simulink library "lib_all"  (Simulink 7.6)
settings: Solver=ode45  SolverMode=SingleTasking  StartTime=0.0  StopTime=10.0  RelTol=1e-3
values from neighbouring files (symbols this model uses):
  map_lib_03 = [6.8679 7.0159 7.4571 8.1849 9.1892 10.456 11.97 13.71 15.655 17.781 20.063 22.474 ... (803 elements, 73x11)]    [scr_simdata.mat]    (also in scr_simdata_all.mat)
  map_lib_06 = [2.5784 2.6727 2.9588 3.4453 4.1444 5.068 6.226 7.6242 9.2634 11.139 13.24 15.552 ... (803 elements, 73x11)]    [scr_simdata.mat]    (also in scr_simdata_all.mat)
  map_lib_09 = [0.0025784 0.0026727 0.0029588 0.0034453 0.0041444 0.005068 0.006226 0.0076242 0.0092634 0.011139 0.01324 0.015552 ... (803 elements, 73x11)]    [scr_simdata.mat]    (also in scr_simdata_all.mat)
  map_lib_10 = [2.74 2.75 2.76 2.77 2.78 2.79 2.8 2.81 2.82 2.83 2.84 2.85 ... (81 elements, 1x81)]    [scr_simdata.mat]    (also in scr_simdata_all.mat)
  map_lib_11 = [1.1983 1.2027 1.207 1.2113 1.2156 1.2198 1.2241 1.2283 1.2326 1.2368 1.241 1.2451 ... (81 elements, 1x81)]    [scr_simdata.mat]    (also in scr_simdata_all.mat)
  map_lib_12 = [2.74 2.75 2.76 2.77 2.78 2.79 2.8 2.81 2.82 2.83 2.84 2.85 ... (81 elements, 1x81)]    [scr_simdata.mat]    (also in scr_simdata_all.mat)
  map_lib_13 = [1.74 1.75 1.76 1.77 1.78 1.79 1.8 1.81 1.82 1.83 1.84 1.85 ... (81 elements, 1x81)]    [scr_simdata.mat]    (also in scr_simdata_all.mat)
  map_lib_16 = [0.00048828125 1.6159 1.8962 2.0821 2.2251 2.3426 2.4433 2.5318 2.611 2.683 2.749 2.8101 ... (1419 elements, 129x11)]    [scr_simdata.mat]    (also in scr_simdata_all.mat)
  map_mr2_01 = [0 800 1600 2400 3200 4000]    [scr_simdata.mat]    (also in scr_simdata_all.mat)
  map_mr2_02 = [1.32 1.32 1.33 1.34 1.36 1.38]    [scr_simdata.mat]    (also in scr_simdata_all.mat)
  map_mr2_03 = [0 800 1600 2400 3200 4000]    [scr_simdata.mat]    (also in scr_simdata_all.mat)
  map_mr2_04 = [1.32 1.32 1.33 1.34 1.36 1.38]    [scr_simdata.mat]    (also in scr_simdata_all.mat)
  map_mr2_05 = [0 800 1600 2400 3200 4000]    [scr_simdata.mat]    (also in scr_simdata_all.mat)
  map_mr2_06 = [1.32 1.32 1.33 1.34 1.36 1.38]    [scr_simdata.mat]    (also in scr_simdata_all.mat)
  Parameter1 = 6    [scr_simdata.mat]    (also in scr_simdata_all.mat)
  Parameter101 = 30    [scr_simdata.mat]    (also in scr_simdata_all.mat)
  Parameter102 = 12    [scr_simdata.mat]    (also in scr_simdata_all.mat)
  Parameter103 = -240    [scr_simdata.mat]    (also in scr_simdata_all.mat)
  Parameter108 = 48    [scr_simdata.mat]    (also in scr_simdata_all.mat)
  Parameter120 = 16    [scr_simdata.mat]    (also in scr_simdata_all.mat)
  Parameter121 = 87    [scr_simdata.mat]    (also in scr_simdata_all.mat)
  Parameter123 = 78    [scr_simdata.mat]    (also in scr_simdata_all.mat)
  Parameter124 = 60    [scr_simdata.mat]    (also in scr_simdata_all.mat)
  Parameter125 = -480    [scr_simdata.mat]    (also in scr_simdata_all.mat)
  Parameter130 = 96    [scr_simdata.mat]    (also in scr_simdata_all.mat)
  Parameter131 = 3    [scr_simdata.mat]    (also in scr_simdata_all.mat)
  Parameter144 = 3    [scr_simdata.mat]    (also in scr_simdata_all.mat)
  Parameter18 = 128    [scr_simdata.mat]    (also in scr_simdata_all.mat)
  Parameter19 = 16    [scr_simdata.mat]    (also in scr_simdata_all.mat)
  Parameter2 = 12    [scr_simdata.mat]    (also in scr_simdata_all.mat)
  Parameter20 = 7    [scr_simdata.mat]    (also in scr_simdata_all.mat)
  Parameter21 = 4    [scr_simdata.mat]    (also in scr_simdata_all.mat)
  Parameter3 = 18    [scr_simdata.mat]    (also in scr_simdata_all.mat)
  Parameter32 = 1    [scr_simdata.mat]    (also in scr_simdata_all.mat)
  Parameter34 = 24    [scr_simdata.mat]    (also in scr_simdata_all.mat)
  Parameter4 = 138    [scr_simdata.mat]    (also in scr_simdata_all.mat)
  Parameter42 = 48    [scr_simdata.mat]    (also in scr_simdata_all.mat)
  Parameter5 = 135    [scr_simdata.mat]    (also in scr_simdata_all.mat)
  Parameter55 = 0    [scr_simdata.mat]    (also in scr_simdata_all.mat)
  Parameter56 = 0    [scr_simdata.mat]    (also in scr_simdata_all.mat)
  Parameter57 = 1    [scr_simdata.mat]    (also in scr_simdata_all.mat)
  Parameter58 = 1    [scr_simdata.mat]    (also in scr_simdata_all.mat)
  Parameter59 = 2    [scr_simdata.mat]    (also in scr_simdata_all.mat)
  Parameter6 = 132    [scr_simdata.mat]    (also in scr_simdata_all.mat)
  Parameter60 = 2    [scr_simdata.mat]    (also in scr_simdata_all.mat)
  Parameter63 = 144    [scr_simdata.mat]    (also in scr_simdata_all.mat)
  Parameter7 = 126    [scr_simdata.mat]    (also in scr_simdata_all.mat)
  Parameter76 = 64    [scr_simdata.mat]    (also in scr_simdata_all.mat)
  Parameter77 = 135    [scr_simdata.mat]    (also in scr_simdata_all.mat)
  Parameter79 = 126    [scr_simdata.mat]    (also in scr_simdata_all.mat)
  Parameter80 = 108    [scr_simdata.mat]    (also in scr_simdata_all.mat)
  Parameter81 = 0    [scr_simdata.mat]    (also in scr_simdata_all.mat)
  Parameter86 = 0    [scr_simdata.mat]    (also in scr_simdata_all.mat)
  Parameter98 = 112    [scr_simdata.mat]    (also in scr_simdata_all.mat)
  Parameter99 = 39    [scr_simdata.mat]    (also in scr_simdata_all.mat)
undefined symbols (not defined in the file or in its folder): cylvolkp_hih_map2h, cylvolkp_map2h, pk1k_map2h
libraries: simulink x2
(part 23 of 36)

== system lib_all/SubSystem800/SubSystem819/SubSystem846/SubSystem854/SubSystem857 ==
  note: ***編集禁止***
  In1 : Inport
  In2 : Inport  Port=2
  RO1 : RelationalOperator  Operator===  InputSameDT=on
  single1 : DataTypeConversion  OutDataTypeStr=single  SaturateOnIntegerOverflow=on  RndMeth=Zero
  single2 : DataTypeConversion  OutDataTypeStr=single  SaturateOnIntegerOverflow=on  RndMeth=Zero
  Out1 : Outport
  wires:
  RO1/1 -> Out1/1
  In2/1 -> single2/1
  In1/1 -> single1/1
  single1/1 -> RO1/1
  single2/1 -> RO1/2

== system lib_all/SubSystem800/SubSystem819/SubSystem846/SubSystem854/SubSystem858 ==
  Block2180 : Inport
  Block2181 : Inport  Port=2
  Block2182 : EnablePort
  Block2183 : Demux  Outputs=2
  Block2184 : Mux  Inputs=2
  SubSystem859 : Subsystem
  SubSystem863 : Subsystem
  Block2193 : Outport
  wires:
  Block2180/1 -> Block2183/1
  Block2181/1 -> SubSystem863/1(Block2189), SubSystem859/1(Block2185)
  Block2183/1 -> SubSystem859/2(Block2186)
  Block2183/2 -> SubSystem863/2(Block2190)
  SubSystem859/1(Block2188) -> Block2184/1
  SubSystem863/1(Block2192) -> Block2184/2
  Block2184/1 -> Block2193/1

== system lib_all/SubSystem800/SubSystem819/SubSystem846/SubSystem854/SubSystem867 ==
  Block2194 : Inport
  Block2195 : Inport  Port=2
  Block2196 : EnablePort
  Block2197 : Demux  Outputs=2
  Block2198 : Mux  Inputs=2
  Block2199 : Sum  OutDataTypeStr=double  RndMeth=Zero  SaturateOnIntegerOverflow=on  InputSameDT=on
  SubSystem868 : Subsystem  TreatAsAtomicUnit=on  mask(***ATC.DSRVEC***){bd_version=1.01.A, r0=DSM4, r1=-1, r2=, r3=, r4=, r5=, r6=, r7=, r8=, r9=, c0=, c1=, c2=, c3=, c4=, c5=, c6=, c7=, c8=, c9=}
      mask prompts: bd_version "Version"
      mask help: これはAutoCからのブロックです．編集禁止．
      settings: OpenFcn=warndlg('変更禁止!');
      mask Help: web(ui_blockhelpmng(gcb));
      mask Display: disp('ATC.DSRVEC')
  SubSystem869 : Subsystem
  SubSystem873 : Subsystem
  Block2208 : Outport
  wires:
  Block2197/2 -> SubSystem873/2(Block2205)
  Block2197/1 -> SubSystem869/2(Block2201)
  Block2194/1 -> Block2197/1
  SubSystem869/1(Block2203) -> Block2198/1
  SubSystem873/1(Block2207) -> Block2198/2
  Block2199/1 -> Block2208/1
  SubSystem868/1(Output) -> Block2199/1
  Block2198/1 -> Block2199/2
  Block2195/1 -> SubSystem873/1(Block2204), SubSystem869/1(Block2200), SubSystem868/1(element)

== system lib_all/SubSystem800/SubSystem819/SubSystem846/SubSystem854/SubSystem877 ==
  Block2209 : Inport
  Block2210 : Inport  Port=2
  Block2211 : EnablePort
  Block2212 : Demux  Outputs=2
  Block2213 : Mux  Inputs=2
  Block2214 : Sum  OutDataTypeStr=double  RndMeth=Zero  SaturateOnIntegerOverflow=on  InputSameDT=on
  SubSystem878 : Subsystem  TreatAsAtomicUnit=on  mask(***ATC.DSRVEC***){bd_version=1.01.A, r0=DSM2, r1=-1, r2=, r3=, r4=, r5=, r6=, r7=, r8=, r9=, c0=, c1=, c2=, c3=, c4=, c5=, c6=, c7=, c8=, c9=}
      mask prompts: bd_version "Version"
      mask help: これはAutoCからのブロックです．編集禁止．
      settings: OpenFcn=warndlg('変更禁止!');
      mask Help: web(ui_blockhelpmng(gcb));
      mask Display: disp('ATC.DSRVEC')
  SubSystem879 : Subsystem
  SubSystem883 : Subsystem
  Block2223 : Outport
  wires:
  Block2209/1 -> Block2212/1
  Block2212/1 -> SubSystem879/2(Block2216)
  Block2212/2 -> SubSystem883/2(Block2220)
  SubSystem879/1(Block2218) -> Block2213/1
  SubSystem883/1(Block2222) -> Block2213/2
  Block2214/1 -> Block2223/1
  Block2213/1 -> Block2214/2
  Block2210/1 -> SubSystem883/1(Block2219), SubSystem879/1(Block2215), SubSystem878/1(element)
  SubSystem878/1(Output) -> Block2214/1

== system lib_all/SubSystem800/SubSystem887/SubSystem900/SubSystem903/SubSystem904 ==
  Block2310 : Inport
  Block2311 : Inport  Port=2
  Block2312 : Constant  Value=Parameter32  OutDataTypeStr=double  SampleTime=-1
  Block2313 : Constant  Value=2  OutDataTypeStr=double  SampleTime=-1
  Block2314 : Constant  Value=Parameter42  OutDataTypeStr=double  SampleTime=-1
  Block2315 : Constant  Value=0  OutDataTypeStr=double  SampleTime=-1
  Block2316 : Constant  Value=72  OutDataTypeStr=double  SampleTime=-1
  Block2317 : Constant  Value=Parameter34  OutDataTypeStr=double  SampleTime=-1
  Block2318 : Logic  Operator=OR
  Block2319 : Sum  Inputs=+-  OutDataTypeStr=double  RndMeth=Zero  SaturateOnIntegerOverflow=on  InputSameDT=on
  Block2320 : Switch  Threshold=0.5  RndMeth=Zero  SaturateOnIntegerOverflow=on  InputSameDT=on
  Block2321 : Switch  Threshold=0.5  RndMeth=Zero  SaturateOnIntegerOverflow=on  InputSameDT=on
  SubSystem905 : Subsystem
  SubSystem906 : Subsystem
  SubSystem907 : Subsystem
      settings: RTWSystemCode=Inline
  Block2328 : Outport
  wires:
  Block2321/1 -> Block2328/1
  Block2320/1 -> Block2319/2
  Block2317/1 -> Block2320/3
  Block2314/1 -> Block2320/1
  Block2318/1 -> Block2321/2
  SubSystem906/1(Out1) -> Block2318/2
  SubSystem905/1(Out1) -> Block2320/2, Block2318/1
  Block2313/1 -> SubSystem906/2(In2)
  Block2312/1 -> SubSystem905/1(In1)
  Block2311/1 -> SubSystem906/1(In1), SubSystem905/2(In2)
  Block2310/1 -> Block2319/1, Block2321/3
  Block2319/1 -> SubSystem907/2(Block2323)
  Block2315/1 -> SubSystem907/3(Block2324)
  Block2316/1 -> SubSystem907/1(Block2322)
  SubSystem907/1(Block2327) -> Block2321/1

== system lib_all/SubSystem800/SubSystem887/SubSystem900/SubSystem903/SubSystem909 ==
  Block2329 : Inport
  Block2330 : Inport  Port=2
  Block2331 : Inport  Port=3
  Block2332 : Sum  OutDataTypeStr=double  RndMeth=Zero  SaturateOnIntegerOverflow=on  InputSameDT=on
  Block2333 : Switch  Threshold=0.5  RndMeth=Zero  SaturateOnIntegerOverflow=on  InputSameDT=on
  SubSystem910 : Subsystem
  Block2334 : Outport
  wires:
  Block2329/1 -> Block2332/1
  Block2333/1 -> Block2334/1
  Block2332/1 -> Block2333/1
  SubSystem910/1(Out1) -> Block2333/2
  Block2331/1 -> SubSystem910/2(In2)
  Block2330/1 -> Block2332/2, Block2333/3, SubSystem910/1(In1)

== system lib_all/SubSystem800/SubSystem887/SubSystem900/SubSystem912/SubSystem913 ==
  note: ***編集禁止***
  In1 : Inport
  In2 : Inport  Port=2
  RO1 : RelationalOperator  Operator=>  InputSameDT=on
  single1 : DataTypeConversion  OutDataTypeStr=single  SaturateOnIntegerOverflow=on  RndMeth=Zero
  single2 : DataTypeConversion  OutDataTypeStr=single  SaturateOnIntegerOverflow=on  RndMeth=Zero
  Out1 : Outport
  wires:
  RO1/1 -> Out1/1
  In2/1 -> single2/1
  In1/1 -> single1/1
  single1/1 -> RO1/1
  single2/1 -> RO1/2

== system lib_all/SubSystem800/SubSystem887/SubSystem914/SubSystem916/SubSystem917 ==
  note: ***編集禁止***
  mult : Inport
  div : Inport  Port=2
  init : Inport  Port=3
  Product : Subsystem  TreatAsAtomicUnit=on
      settings: RTWSystemCode=Inline
  Out : Outport
  wires:
  Product/1(Out) -> Out/1
  mult/1 -> Product/1(mult)
  div/1 -> Product/2(div)
  init/1 -> Product/3(init)

== system lib_all/SubSystem800/SubSystem887/SubSystem914/SubSystem916/SubSystem918 ==
  note: ***編集禁止***
  element : Inport
  Inside_DSR : DataStoreRead  DataStoreName=DSM95
  Selector : Selector  IndexOptions=Index vector (port)  Indices=[1 3]  OutputSizes=1
  Output : Outport
  wires:
  Selector/1 -> Output/1
  element/1 -> Selector/2
  Inside_DSR/1 -> Selector/1

== system lib_all/SubSystem800/SubSystem887/SubSystem914/SubSystem916/SubSystem919 ==
  Block2377 : Inport
  Block2378 : Inport  Port=2
  Block2379 : Inport  Port=3
  Block2380 : Sum  OutDataTypeStr=double  RndMeth=Zero  SaturateOnIntegerOverflow=on  InputSameDT=on
  Block2381 : Sum  Inputs=+-  OutDataTypeStr=double  RndMeth=Zero  SaturateOnIntegerOverflow=on  InputSameDT=on
  Block2382 : Switch  Threshold=0.5  RndMeth=Zero  SaturateOnIntegerOverflow=on  InputSameDT=on
  Block2383 : Switch  Threshold=0.5  RndMeth=Zero  SaturateOnIntegerOverflow=on  InputSameDT=on
  SubSystem920 : Subsystem
  SubSystem921 : Subsystem
  Block2384 : Outport
  wires:
  Block2378/1 -> SubSystem920/1(In1), Block2381/1, SubSystem921/1(In1), Block2383/3, Block2380/2
  Block2377/1 -> Block2380/1, Block2381/2, SubSystem920/2(In2)
  SubSystem920/1(Out1) -> Block2382/2
  Block2379/1 -> SubSystem921/2(In2)
  SubSystem921/1(Out1) -> Block2383/2
  Block2383/1 -> Block2382/3
  Block2380/1 -> Block2383/1
  Block2382/1 -> Block2384/1
  Block2381/1 -> Block2382/1

== system lib_all/SubSystem800/SubSystem887/SubSystem914/SubSystem922/SubSystem923 ==
  note: ***編集禁止***
  In1 : Inport
  In2 : Inport  Port=2
  LO1 : Logic
  RO1 : RelationalOperator  Operator=<  InputSameDT=on
  RO2 : RelationalOperator  Operator=~=  InputSameDT=on
  single1 : DataTypeConversion  OutDataTypeStr=single  SaturateOnIntegerOverflow=on  RndMeth=Zero
  single2 : DataTypeConversion  OutDataTypeStr=single  SaturateOnIntegerOverflow=on  RndMeth=Zero
  Out1 : Outport
  wires:
  LO1/1 -> Out1/1
  RO2/1 -> LO1/2
  RO1/1 -> LO1/1
  In2/1 -> single2/1
  In1/1 -> single1/1
  single1/1 -> RO2/1, RO1/1
  single2/1 -> RO2/2, RO1/2

== system lib_all/SubSystem800/SubSystem887/SubSystem914/SubSystem922/SubSystem924 ==
  note: ***編集禁止***
  In1 : Inport
  In2 : Inport  Port=2
  RO1 : RelationalOperator  Operator===  InputSameDT=on
  single1 : DataTypeConversion  OutDataTypeStr=single  SaturateOnIntegerOverflow=on  RndMeth=Zero
  single2 : DataTypeConversion  OutDataTypeStr=single  SaturateOnIntegerOverflow=on  RndMeth=Zero
  Out1 : Outport
  wires:
  RO1/1 -> Out1/1
  In2/1 -> single2/1
  In1/1 -> single1/1
  single1/1 -> RO1/1
  single2/1 -> RO1/2

== system lib_all/SubSystem800/SubSystem887/SubSystem914/SubSystem922/SubSystem925 ==
  note: ***編集禁止***
  In1 : Inport
  In2 : Inport  Port=2
  RO1 : RelationalOperator  Operator===  InputSameDT=on
  single1 : DataTypeConversion  OutDataTypeStr=single  SaturateOnIntegerOverflow=on  RndMeth=Zero
  single2 : DataTypeConversion  OutDataTypeStr=single  SaturateOnIntegerOverflow=on  RndMeth=Zero
  Out1 : Outport
  wires:
  RO1/1 -> Out1/1
  In2/1 -> single2/1
  In1/1 -> single1/1
  single1/1 -> RO1/1
  single2/1 -> RO1/2

== system lib_all/SubSystem800/SubSystem887/SubSystem914/SubSystem922/SubSystem926 ==
  Block2405 : Inport
  Block2406 : Inport  Port=2
  Block2407 : EnablePort
  Block2408 : Demux  Outputs=2
  Block2409 : Mux  Inputs=2
  SubSystem927 : Subsystem
  SubSystem931 : Subsystem
  Block2418 : Outport
  wires:
  Block2405/1 -> Block2408/1
  Block2406/1 -> SubSystem931/1(Block2414), SubSystem927/1(Block2410)
  Block2408/1 -> SubSystem927/2(Block2411)
  Block2408/2 -> SubSystem931/2(Block2415)
  SubSystem927/1(Block2413) -> Block2409/1
  SubSystem931/1(Block2417) -> Block2409/2
  Block2409/1 -> Block2418/1

== system lib_all/SubSystem800/SubSystem887/SubSystem914/SubSystem922/SubSystem935 ==
  Block2419 : Inport
  Block2420 : Inport  Port=2
  Block2421 : EnablePort
  Block2422 : Demux  Outputs=2
  Block2423 : Mux  Inputs=2
  Block2424 : Sum  OutDataTypeStr=double  RndMeth=Zero  SaturateOnIntegerOverflow=on  InputSameDT=on
  SubSystem936 : Subsystem  TreatAsAtomicUnit=on  mask(***ATC.DSRVEC***){bd_version=1.01.A, r0=DSM4, r1=-1, r2=, r3=, r4=, r5=, r6=, r7=, r8=, r9=, c0=, c1=, c2=, c3=, c4=, c5=, c6=, c7=, c8=, c9=}
      mask prompts: bd_version "Version"
      mask help: これはAutoCからのブロックです．編集禁止．
      settings: OpenFcn=warndlg('変更禁止!');
      mask Help: web(ui_blockhelpmng(gcb));
      mask Display: disp('ATC.DSRVEC')
  SubSystem937 : Subsystem
  SubSystem941 : Subsystem
  Block2433 : Outport
  wires:
  Block2422/2 -> SubSystem941/2(Block2430)
  Block2422/1 -> SubSystem937/2(Block2426)
  Block2419/1 -> Block2422/1
  SubSystem937/1(Block2428) -> Block2423/1
  SubSystem941/1(Block2432) -> Block2423/2
  Block2424/1 -> Block2433/1
  SubSystem936/1(Output) -> Block2424/1
  Block2423/1 -> Block2424/2
  Block2420/1 -> SubSystem941/1(Block2429), SubSystem937/1(Block2425), SubSystem936/1(element)

== system lib_all/SubSystem800/SubSystem887/SubSystem914/SubSystem922/SubSystem945 ==
  Block2434 : Inport
  Block2435 : Inport  Port=2
  Block2436 : EnablePort
  Block2437 : Demux  Outputs=2
  Block2438 : Mux  Inputs=2
  Block2439 : Sum  OutDataTypeStr=double  RndMeth=Zero  SaturateOnIntegerOverflow=on  InputSameDT=on
  SubSystem946 : Subsystem  TreatAsAtomicUnit=on  mask(***ATC.DSRVEC***){bd_version=1.01.A, r0=DSM2, r1=-1, r2=, r3=, r4=, r5=, r6=, r7=, r8=, r9=, c0=, c1=, c2=, c3=, c4=, c5=, c6=, c7=, c8=, c9=}
      mask prompts: bd_version "Version"
      mask help: これはAutoCからのブロックです．編集禁止．
      settings: OpenFcn=warndlg('変更禁止!');
      mask Help: web(ui_blockhelpmng(gcb));
      mask Display: disp('ATC.DSRVEC')
  SubSystem947 : Subsystem
  SubSystem951 : Subsystem
  Block2448 : Outport
  wires:
  Block2434/1 -> Block2437/1
  Block2437/1 -> SubSystem947/2(Block2441)
  Block2437/2 -> SubSystem951/2(Block2445)
  SubSystem947/1(Block2443) -> Block2438/1
  SubSystem951/1(Block2447) -> Block2438/2
  Block2439/1 -> Block2448/1
  Block2438/1 -> Block2439/2
  Block2435/1 -> SubSystem951/1(Block2444), SubSystem947/1(Block2440), SubSystem946/1(element)
  SubSystem946/1(Output) -> Block2439/1

== system lib_all/SubSystem959/SubSystem963/SubSystem965/SubSystem968/SubSystem969 ==
  note: ***編集禁止***
  mult : Inport
  div : Inport  Port=2
  init : Inport  Port=3
  Product : Subsystem  TreatAsAtomicUnit=on
      settings: RTWSystemCode=Inline
  Out : Outport
  wires:
  init/1 -> Product/3(init)
  div/1 -> Product/2(div)
  mult/1 -> Product/1(mult)
  Product/1(Out) -> Out/1

== system lib_all/SubSystem959/SubSystem963/SubSystem965/SubSystem968/SubSystem970 ==
  note: ***編集禁止***
  element : Inport
  Inside_DSR : DataStoreRead  DataStoreName=DSM95
  Selector : Selector  IndexOptions=Index vector (port)  Indices=[1 3]  OutputSizes=1
  Output : Outport
  wires:
  Inside_DSR/1 -> Selector/1
  element/1 -> Selector/2
  Selector/1 -> Output/1

== system lib_all/SubSystem959/SubSystem963/SubSystem965/SubSystem968/SubSystem971 ==
  Block2520 : Inport
  Block2521 : Inport  Port=2
  Block2522 : Inport  Port=3
  Block2523 : Sum  OutDataTypeStr=double  RndMeth=Zero  SaturateOnIntegerOverflow=on  InputSameDT=on
  Block2524 : Sum  Inputs=+-  OutDataTypeStr=double  RndMeth=Zero  SaturateOnIntegerOverflow=on  InputSameDT=on
  Block2525 : Switch  Threshold=0.5  RndMeth=Zero  SaturateOnIntegerOverflow=on  InputSameDT=on
  Block2526 : Switch  Threshold=0.5  RndMeth=Zero  SaturateOnIntegerOverflow=on  InputSameDT=on
  SubSystem972 : Subsystem
  SubSystem973 : Subsystem
  Block2527 : Outport
  wires:
  Block2524/1 -> Block2525/1
  Block2525/1 -> Block2527/1
  Block2523/1 -> Block2526/1
  Block2526/1 -> Block2525/3
  SubSystem973/1(Out1) -> Block2526/2
  Block2522/1 -> SubSystem973/2(In2)
  SubSystem972/1(Out1) -> Block2525/2
  Block2520/1 -> SubSystem972/2(In2), Block2524/2, Block2523/1
  Block2521/1 -> Block2523/2, Block2526/3, SubSystem973/1(In1), Block2524/1, SubSystem972/1(In1)

== system lib_all/SubSystem959/SubSystem963/SubSystem965/SubSystem974/SubSystem1008 ==
  Block2594 : Inport
  Block2595 : Inport  Port=2
  Block2596 : EnablePort
  Block2597 : Demux  Outputs=2
  Block2598 : Mux  Inputs=2
  Block2599 : Sum  OutDataTypeStr=double  RndMeth=Zero  SaturateOnIntegerOverflow=on  InputSameDT=on
  SubSystem1009 : Subsystem  TreatAsAtomicUnit=on  mask(***ATC.DSRVEC***){bd_version=1.01.A, r0=DSM3, r1=-1, r2=, r3=, r4=, r5=, r6=, r7=, r8=, r9=, c0=, c1=, c2=, c3=, c4=, c5=, c6=, c7=, c8=, c9=}
      mask prompts: bd_version "Version"
      mask help: これはAutoCからのブロックです．編集禁止．
      settings: OpenFcn=warndlg('変更禁止!');
      mask Help: web(ui_blockhelpmng(gcb));
      mask Display: disp('ATC.DSRVEC')
  SubSystem1010 : Subsystem
  SubSystem1014 : Subsystem
  Block2608 : Outport
  wires:
  Block2595/1 -> SubSystem1009/1(element), SubSystem1010/1(Block2600), SubSystem1014/1(Block2604)
  Block2594/1 -> Block2597/1
  Block2597/1 -> SubSystem1010/2(Block2601)
  Block2597/2 -> SubSystem1014/2(Block2605)
  SubSystem1010/1(Block2603) -> Block2598/1
  SubSystem1014/1(Block2607) -> Block2598/2
  Block2599/1 -> Block2608/1
  SubSystem1009/1(Output) -> Block2599/1
  Block2598/1 -> Block2599/2

== system lib_all/SubSystem959/SubSystem963/SubSystem965/SubSystem974/SubSystem975 ==
  note: ***編集禁止***
  In1 : Inport
  In2 : Inport  Port=2
  LO1 : Logic
  RO1 : RelationalOperator  Operator=<  InputSameDT=on
  RO2 : RelationalOperator  Operator=~=  InputSameDT=on
  single1 : DataTypeConversion  OutDataTypeStr=single  SaturateOnIntegerOverflow=on  RndMeth=Zero
  single2 : DataTypeConversion  OutDataTypeStr=single  SaturateOnIntegerOverflow=on  RndMeth=Zero
  Out1 : Outport
  wires:
  single2/1 -> RO1/2, RO2/2
  single1/1 -> RO1/1, RO2/1
  In1/1 -> single1/1
  In2/1 -> single2/1
  RO1/1 -> LO1/1
  RO2/1 -> LO1/2
  LO1/1 -> Out1/1

== system lib_all/SubSystem959/SubSystem963/SubSystem965/SubSystem974/SubSystem976 ==
  note: ***編集禁止***
  In1 : Inport
  In2 : Inport  Port=2
  RO1 : RelationalOperator  Operator===  InputSameDT=on
  single1 : DataTypeConversion  OutDataTypeStr=single  SaturateOnIntegerOverflow=on  RndMeth=Zero
  single2 : DataTypeConversion  OutDataTypeStr=single  SaturateOnIntegerOverflow=on  RndMeth=Zero
  Out1 : Outport
  wires:
  single2/1 -> RO1/2
  single1/1 -> RO1/1
  In1/1 -> single1/1
  In2/1 -> single2/1
  RO1/1 -> Out1/1

== system lib_all/SubSystem959/SubSystem963/SubSystem965/SubSystem974/SubSystem977 ==
  note: ***編集禁止***
  In1 : Inport
  In2 : Inport  Port=2
  RO1 : RelationalOperator  Operator===  InputSameDT=on
  single1 : DataTypeConversion  OutDataTypeStr=single  SaturateOnIntegerOverflow=on  RndMeth=Zero
  single2 : DataTypeConversion  OutDataTypeStr=single  SaturateOnIntegerOverflow=on  RndMeth=Zero
  Out1 : Outport
  wires:
  single2/1 -> RO1/2
  single1/1 -> RO1/1
  In1/1 -> single1/1
  In2/1 -> single2/1
  RO1/1 -> Out1/1

== system lib_all/SubSystem959/SubSystem963/SubSystem965/SubSystem974/SubSystem978 ==
  note: ***編集禁止***
  In1 : Inport
  In2 : Inport  Port=2
  RO1 : RelationalOperator  Operator===  InputSameDT=on
  single1 : DataTypeConversion  OutDataTypeStr=single  SaturateOnIntegerOverflow=on  RndMeth=Zero
  single2 : DataTypeConversion  OutDataTypeStr=single  SaturateOnIntegerOverflow=on  RndMeth=Zero
  Out1 : Outport
  wires:
  single2/1 -> RO1/2
  single1/1 -> RO1/1
  In1/1 -> single1/1
  In2/1 -> single2/1
  RO1/1 -> Out1/1

== system lib_all/SubSystem959/SubSystem963/SubSystem965/SubSystem974/SubSystem979 ==
  Block2550 : Inport
  Block2551 : Inport  Port=2
  Block2552 : EnablePort
  Block2553 : Demux  Outputs=2
  Block2554 : Mux  Inputs=2
  SubSystem980 : Subsystem
  SubSystem984 : Subsystem
  Block2563 : Outport
  wires:
  Block2554/1 -> Block2563/1
  SubSystem984/1(Block2562) -> Block2554/2
  SubSystem980/1(Block2558) -> Block2554/1
  Block2553/2 -> SubSystem984/2(Block2560)
  Block2553/1 -> SubSystem980/2(Block2556)
  Block2551/1 -> SubSystem980/1(Block2555), SubSystem984/1(Block2559)
  Block2550/1 -> Block2553/1

== system lib_all/SubSystem959/SubSystem963/SubSystem965/SubSystem974/SubSystem988 ==
  Block2564 : Inport
  Block2565 : Inport  Port=2
  Block2566 : EnablePort
  Block2567 : Demux  Outputs=2
  Block2568 : Mux  Inputs=2
  Block2569 : Sum  OutDataTypeStr=double  RndMeth=Zero  SaturateOnIntegerOverflow=on  InputSameDT=on
  SubSystem989 : Subsystem  TreatAsAtomicUnit=on  mask(***ATC.DSRVEC***){bd_version=1.01.A, r0=DSM1, r1=-1, r2=, r3=, r4=, r5=, r6=, r7=, r8=, r9=, c0=, c1=, c2=, c3=, c4=, c5=, c6=, c7=, c8=, c9=}
      mask prompts: bd_version "Version"
      mask help: これはAutoCからのブロックです．編集禁止．
      settings: OpenFcn=warndlg('変更禁止!');
      mask Help: web(ui_blockhelpmng(gcb));
      mask Display: disp('ATC.DSRVEC')
  SubSystem990 : Subsystem
  SubSystem994 : Subsystem
  Block2578 : Outport
  wires:
  Block2565/1 -> SubSystem989/1(element), SubSystem990/1(Block2570), SubSystem994/1(Block2574)
  Block2568/1 -> Block2569/2
  SubSystem989/1(Output) -> Block2569/1
  Block2569/1 -> Block2578/1
  SubSystem994/1(Block2577) -> Block2568/2
  SubSystem990/1(Block2573) -> Block2568/1
  Block2567/2 -> SubSystem994/2(Block2575)
  Block2567/1 -> SubSystem990/2(Block2571)
  Block2564/1 -> Block2567/1

== system lib_all/SubSystem959/SubSystem963/SubSystem965/SubSystem974/SubSystem998 ==
  Block2579 : Inport
  Block2580 : Inport  Port=2
  Block2581 : EnablePort
  Block2582 : Demux  Outputs=2
  Block2583 : Mux  Inputs=2
  Block2584 : Sum  OutDataTypeStr=double  RndMeth=Zero  SaturateOnIntegerOverflow=on  InputSameDT=on
  SubSystem1000 : Subsystem
  SubSystem1004 : Subsystem
  SubSystem999 : Subsystem  TreatAsAtomicUnit=on  mask(***ATC.DSRVEC***){bd_version=1.01.A, r0=DSM5, r1=-1, r2=, r3=, r4=, r5=, r6=, r7=, r8=, r9=, c0=, c1=, c2=, c3=, c4=, c5=, c6=, c7=, c8=, c9=}
      mask prompts: bd_version "Version"
      mask help: これはAutoCからのブロックです．編集禁止．
      settings: OpenFcn=warndlg('変更禁止!');
      mask Help: web(ui_blockhelpmng(gcb));
      mask Display: disp('ATC.DSRVEC')
  Block2593 : Outport
  wires:
  Block2580/1 -> SubSystem999/1(element), SubSystem1000/1(Block2585), SubSystem1004/1(Block2589)
  Block2583/1 -> Block2584/2
  SubSystem999/1(Output) -> Block2584/1
  Block2584/1 -> Block2593/1
  SubSystem1004/1(Block2592) -> Block2583/2
  SubSystem1000/1(Block2588) -> Block2583/1
  Block2582/2 -> SubSystem1004/2(Block2590)
  Block2582/1 -> SubSystem1000/2(Block2586)
  Block2579/1 -> Block2582/1

== system lib_all/SubSystem1023/SubSystem1025/SubSystem1038/SubSystem1041/SubSystem1042/SubSystem1043 ==
  note: ***編集禁止***
  In1 : Inport
  In2 : Inport  Port=2
  RO1 : RelationalOperator  Operator===  InputSameDT=on
  single1 : DataTypeConversion  OutDataTypeStr=single  SaturateOnIntegerOverflow=on  RndMeth=Zero
  single2 : DataTypeConversion  OutDataTypeStr=single  SaturateOnIntegerOverflow=on  RndMeth=Zero
  Out1 : Outport
  wires:
  single2/1 -> RO1/2
  single1/1 -> RO1/1
  In1/1 -> single1/1
  In2/1 -> single2/1
  RO1/1 -> Out1/1

== system lib_all/SubSystem1023/SubSystem1025/SubSystem1038/SubSystem1041/SubSystem1042/SubSystem1044 ==
  note: ***編集禁止***
  In1 : Inport
  In2 : Inport  Port=2
  RO1 : RelationalOperator  Operator===  InputSameDT=on
  single1 : DataTypeConversion  OutDataTypeStr=single  SaturateOnIntegerOverflow=on  RndMeth=Zero
  single2 : DataTypeConversion  OutDataTypeStr=single  SaturateOnIntegerOverflow=on  RndMeth=Zero
  Out1 : Outport
  wires:
  single2/1 -> RO1/2
  single1/1 -> RO1/1
  In1/1 -> single1/1
  In2/1 -> single2/1
  RO1/1 -> Out1/1

== system lib_all/SubSystem1023/SubSystem1025/SubSystem1038/SubSystem1041/SubSystem1042/SubSystem1045 ==
  Block2724 : Inport
  Block2725 : Inport  Port=2
  Block2726 : Inport  Port=3
  Block2727 : Sum  OutDataTypeStr=double  RndMeth=Zero  SaturateOnIntegerOverflow=on  InputSameDT=on
  Block2728 : Switch  Threshold=0.5  RndMeth=Zero  SaturateOnIntegerOverflow=on  InputSameDT=on
  SubSystem1046 : Subsystem
  Block2729 : Outport
  wires:
  Block2725/1 -> SubSystem1046/1(In1), Block2728/3, Block2727/2
  Block2726/1 -> SubSystem1046/2(In2)
  SubSystem1046/1(Out1) -> Block2728/2
  Block2727/1 -> Block2728/1
  Block2728/1 -> Block2729/1
  Block2724/1 -> Block2727/1

== system lib_all/SubSystem1023/SubSystem1025/SubSystem1038/SubSystem1041/SubSystem1047/SubSystem1048 ==
  note: ***編集禁止***
  In1 : Inport
  In2 : Inport  Port=2
  LO1 : Logic
  RO1 : RelationalOperator  Operator=<  InputSameDT=on
  RO2 : RelationalOperator  Operator=~=  InputSameDT=on
  single1 : DataTypeConversion  OutDataTypeStr=single  SaturateOnIntegerOverflow=on  RndMeth=Zero
  single2 : DataTypeConversion  OutDataTypeStr=single  SaturateOnIntegerOverflow=on  RndMeth=Zero
  Out1 : Outport
  wires:
  LO1/1 -> Out1/1
  RO2/1 -> LO1/2
  RO1/1 -> LO1/1
  single2/1 -> RO2/2, RO1/2
  single1/1 -> RO2/1, RO1/1
  In1/1 -> single1/1
  In2/1 -> single2/1

== system lib_all/SubSystem1023/SubSystem1025/SubSystem1052/SubSystem1054/SubSystem1055/Product ==
  note: ***編集禁止***
  mult : Inport
  div : Inport  Port=2
  init : Inport  Port=3
  Constant1 : Constant  OutDataTypeStr=Inherit: Inherit via back propagation
  Constant2 : Constant  Value=0  OutDataTypeStr=Inherit: Inherit via back propagation
  Product : Product  Inputs=*/  SaturateOnIntegerOverflow=on  RndMeth=Zero  InputSameDT=on  OutDataTypeStr=Inherit: Same as first input
  Relational Operator : RelationalOperator  Operator=~=  InputSameDT=on
  Switch : Switch  Threshold=0.5  SaturateOnIntegerOverflow=on  InputSameDT=on
  Switch1 : Switch  Threshold=0.5  SaturateOnIntegerOverflow=on  InputSameDT=on
  Out : Outport
  wires:
  mult/1 -> Product/1
  Product/1 -> Switch/1
  Relational Operator/1 -> Switch/2, Switch1/2
  init/1 -> Switch/3
  Switch/1 -> Out/1
  div/1 -> Switch1/1, Relational Operator/1
  Switch1/1 -> Product/2
  Constant2/1 -> Relational Operator/2
  Constant1/1 -> Switch1/3

== system lib_all/SubSystem1023/SubSystem1025/SubSystem1052/SubSystem1054/SubSystem1057/SubSystem1058 ==
  note: ***編集禁止***
  In1 : Inport
  In2 : Inport  Port=2
  RO1 : RelationalOperator  Operator=>  InputSameDT=on
  single1 : DataTypeConversion  OutDataTypeStr=single  SaturateOnIntegerOverflow=on  RndMeth=Zero
  single2 : DataTypeConversion  OutDataTypeStr=single  SaturateOnIntegerOverflow=on  RndMeth=Zero
  Out1 : Outport
  wires:
  single2/1 -> RO1/2
  single1/1 -> RO1/1
  In1/1 -> single1/1
  In2/1 -> single2/1
  RO1/1 -> Out1/1

== system lib_all/SubSystem1023/SubSystem1025/SubSystem1052/SubSystem1054/SubSystem1057/SubSystem1059 ==
  note: ***編集禁止***
  In1 : Inport
  In2 : Inport  Port=2
  LO1 : Logic
  RO1 : RelationalOperator  Operator=<  InputSameDT=on
  RO2 : RelationalOperator  Operator=~=  InputSameDT=on
  single1 : DataTypeConversion  OutDataTypeStr=single  SaturateOnIntegerOverflow=on  RndMeth=Zero
  single2 : DataTypeConversion  OutDataTypeStr=single  SaturateOnIntegerOverflow=on  RndMeth=Zero
  Out1 : Outport
  wires:
  LO1/1 -> Out1/1
  RO2/1 -> LO1/2
  RO1/1 -> LO1/1
  single2/1 -> RO2/2, RO1/2
  single1/1 -> RO2/1, RO1/1
  In1/1 -> single1/1
  In2/1 -> single2/1

== system lib_all/SubSystem1023/SubSystem1025/SubSystem1052/SubSystem1060/SubSystem1064/SubSystem1065 ==
  Block2812 : Inport
  Block2813 : Inport  Port=2
  Block2814 : MultiPortSwitch  zeroidx=off  RndMeth=Zero  SaturateOnIntegerOverflow=on  InputSameDT=on
  SubSystem1066 : Subsystem  TreatAsAtomicUnit=on  mask(***ATC.DSRVEC***){bd_version=1.01.A, r0=DSM18, r1=-1, r2=, r3=, r4=, r5=, r6=, r7=, r8=, r9=, c0=, c1=, c2=, c3=, c4=, c5=, c6=, c7=, c8=, c9=}
      mask prompts: bd_version "Version"
      mask help: これはAutoCからのブロックです．編集禁止．
      settings: OpenFcn=warndlg('変更禁止!');
      mask Help: web(ui_blockhelpmng(gcb));
      mask Display: disp('ATC.DSRVEC')
  SubSystem1067 : Subsystem  TreatAsAtomicUnit=on  mask(***ATC.DSRVEC***){bd_version=1.01.A, r0=DSM8, r1=-1, r2=, r3=, r4=, r5=, r6=, r7=, r8=, r9=, c0=, c1=, c2=, c3=, c4=, c5=, c6=, c7=, c8=, c9=}
      mask prompts: bd_version "Version"
      mask help: これはAutoCからのブロックです．編集禁止．
      settings: OpenFcn=warndlg('変更禁止!');
      mask Help: web(ui_blockhelpmng(gcb));
      mask Display: disp('ATC.DSRVEC')
  SubSystem1068 : Subsystem  TreatAsAtomicUnit=on  mask(***ATC.DSRVEC***){bd_version=1.01.A, r0=DSM11, r1=-1, r2=, r3=, r4=, r5=, r6=, r7=, r8=, r9=, c0=, c1=, c2=, c3=, c4=, c5=, c6=, c7=, c8=, c9=}
      mask prompts: bd_version "Version"
      mask help: これはAutoCからのブロックです．編集禁止．
      settings: OpenFcn=warndlg('変更禁止!');
      mask Help: web(ui_blockhelpmng(gcb));
      mask Display: disp('ATC.DSRVEC')
  Block2815 : Outport
  wires:
  Block2813/1 -> SubSystem1068/1(element), SubSystem1067/1(element), SubSystem1066/1(element)
  SubSystem1068/1(Output) -> Block2814/4
  SubSystem1067/1(Output) -> Block2814/3
  SubSystem1066/1(Output) -> Block2814/2
  Block2814/1 -> Block2815/1
  Block2812/1 -> Block2814/1

== system lib_all/SubSystem1023/SubSystem1025/SubSystem1052/SubSystem1060/SubSystem1064/SubSystem1069 ==
  Block2816 : Inport
  Block2817 : Inport  Port=2
  Block2818 : MultiPortSwitch  zeroidx=off  RndMeth=Zero  SaturateOnIntegerOverflow=on  InputSameDT=on
  SubSystem1070 : Subsystem  TreatAsAtomicUnit=on  mask(***ATC.DSRVEC***){bd_version=1.01.A, r0=DSM18, r1=-1, r2=, r3=, r4=, r5=, r6=, r7=, r8=, r9=, c0=, c1=, c2=, c3=, c4=, c5=, c6=, c7=, c8=, c9=}
      mask prompts: bd_version "Version"
      mask help: これはAutoCからのブロックです．編集禁止．
      settings: OpenFcn=warndlg('変更禁止!');
      mask Help: web(ui_blockhelpmng(gcb));
      mask Display: disp('ATC.DSRVEC')
  SubSystem1071 : Subsystem  TreatAsAtomicUnit=on  mask(***ATC.DSRVEC***){bd_version=1.01.A, r0=DSM8, r1=-1, r2=, r3=, r4=, r5=, r6=, r7=, r8=, r9=, c0=, c1=, c2=, c3=, c4=, c5=, c6=, c7=, c8=, c9=}
      mask prompts: bd_version "Version"
      mask help: これはAutoCからのブロックです．編集禁止．
      settings: OpenFcn=warndlg('変更禁止!');
      mask Help: web(ui_blockhelpmng(gcb));
      mask Display: disp('ATC.DSRVEC')
  SubSystem1072 : Subsystem  TreatAsAtomicUnit=on  mask(***ATC.DSRVEC***){bd_version=1.01.A, r0=DSM11, r1=-1, r2=, r3=, r4=, r5=, r6=, r7=, r8=, r9=, c0=, c1=, c2=, c3=, c4=, c5=, c6=, c7=, c8=, c9=}
      mask prompts: bd_version "Version"
      mask help: これはAutoCからのブロックです．編集禁止．
      settings: OpenFcn=warndlg('変更禁止!');
      mask Help: web(ui_blockhelpmng(gcb));
      mask Display: disp('ATC.DSRVEC')
  Block2819 : Outport
  wires:
  Block2817/1 -> SubSystem1072/1(element), SubSystem1071/1(element), SubSystem1070/1(element)
  SubSystem1072/1(Output) -> Block2818/4
  SubSystem1071/1(Output) -> Block2818/3
  SubSystem1070/1(Output) -> Block2818/2
  Block2818/1 -> Block2819/1
  Block2816/1 -> Block2818/1

== system lib_all/SubSystem1023/SubSystem1025/SubSystem1052/SubSystem1060/SubSystem1073/SubSystem1074 ==
  note: ***編集禁止***
  element : Inport
  Inside_DSR : DataStoreRead  DataStoreName=DSM4
  Selector : Selector  IndexOptions=Index vector (port)  Indices=[1 3]  OutputSizes=1
  Output : Outport
  wires:
  Inside_DSR/1 -> Selector/1
  element/1 -> Selector/2
  Selector/1 -> Output/1

== system lib_all/SubSystem1023/SubSystem1025/SubSystem1052/SubSystem1060/SubSystem1073/SubSystem1075 ==
  Block2827 : Inport
  Block2828 : Inport  Port=2
  Block2829 : MultiPortSwitch  zeroidx=off  RndMeth=Zero  SaturateOnIntegerOverflow=on  InputSameDT=on
  SubSystem1076 : Subsystem  TreatAsAtomicUnit=on  mask(***ATC.DSRVEC***){bd_version=1.01.A, r0=DSM18, r1=-1, r2=, r3=, r4=, r5=, r6=, r7=, r8=, r9=, c0=, c1=, c2=, c3=, c4=, c5=, c6=, c7=, c8=, c9=}
      mask prompts: bd_version "Version"
      mask help: これはAutoCからのブロックです．編集禁止．
      settings: OpenFcn=warndlg('変更禁止!');
      mask Help: web(ui_blockhelpmng(gcb));
      mask Display: disp('ATC.DSRVEC')
  SubSystem1077 : Subsystem  TreatAsAtomicUnit=on  mask(***ATC.DSRVEC***){bd_version=1.01.A, r0=DSM8, r1=-1, r2=, r3=, r4=, r5=, r6=, r7=, r8=, r9=, c0=, c1=, c2=, c3=, c4=, c5=, c6=, c7=, c8=, c9=}
      mask prompts: bd_version "Version"
      mask help: これはAutoCからのブロックです．編集禁止．
      settings: OpenFcn=warndlg('変更禁止!');
      mask Help: web(ui_blockhelpmng(gcb));
      mask Display: disp('ATC.DSRVEC')
  SubSystem1078 : Subsystem  TreatAsAtomicUnit=on  mask(***ATC.DSRVEC***){bd_version=1.01.A, r0=DSM11, r1=-1, r2=, r3=, r4=, r5=, r6=, r7=, r8=, r9=, c0=, c1=, c2=, c3=, c4=, c5=, c6=, c7=, c8=, c9=}
      mask prompts: bd_version "Version"
      mask help: これはAutoCからのブロックです．編集禁止．
      settings: OpenFcn=warndlg('変更禁止!');
      mask Help: web(ui_blockhelpmng(gcb));
      mask Display: disp('ATC.DSRVEC')
  Block2830 : Outport
  wires:
  Block2828/1 -> SubSystem1078/1(element), SubSystem1077/1(element), SubSystem1076/1(element)
  SubSystem1078/1(Output) -> Block2829/4
  SubSystem1077/1(Output) -> Block2829/3
  SubSystem1076/1(Output) -> Block2829/2
  Block2829/1 -> Block2830/1
  Block2827/1 -> Block2829/1

== system lib_all/SubSystem1023/SubSystem1025/SubSystem1052/SubSystem1060/SubSystem1073/SubSystem1079 ==
  Block2831 : Inport
  Block2832 : Inport  Port=2
  Block2833 : MultiPortSwitch  zeroidx=off  RndMeth=Zero  SaturateOnIntegerOverflow=on  InputSameDT=on
  SubSystem1080 : Subsystem  TreatAsAtomicUnit=on  mask(***ATC.DSRVEC***){bd_version=1.01.A, r0=DSM18, r1=-1, r2=, r3=, r4=, r5=, r6=, r7=, r8=, r9=, c0=, c1=, c2=, c3=, c4=, c5=, c6=, c7=, c8=, c9=}
      mask prompts: bd_version "Version"
      mask help: これはAutoCからのブロックです．編集禁止．
      settings: OpenFcn=warndlg('変更禁止!');
      mask Help: web(ui_blockhelpmng(gcb));
      mask Display: disp('ATC.DSRVEC')
  SubSystem1081 : Subsystem  TreatAsAtomicUnit=on  mask(***ATC.DSRVEC***){bd_version=1.01.A, r0=DSM8, r1=-1, r2=, r3=, r4=, r5=, r6=, r7=, r8=, r9=, c0=, c1=, c2=, c3=, c4=, c5=, c6=, c7=, c8=, c9=}
      mask prompts: bd_version "Version"
      mask help: これはAutoCからのブロックです．編集禁止．
      settings: OpenFcn=warndlg('変更禁止!');
      mask Help: web(ui_blockhelpmng(gcb));
      mask Display: disp('ATC.DSRVEC')
  SubSystem1082 : Subsystem  TreatAsAtomicUnit=on  mask(***ATC.DSRVEC***){bd_version=1.01.A, r0=DSM11, r1=-1, r2=, r3=, r4=, r5=, r6=, r7=, r8=, r9=, c0=, c1=, c2=, c3=, c4=, c5=, c6=, c7=, c8=, c9=}
      mask prompts: bd_version "Version"
      mask help: これはAutoCからのブロックです．編集禁止．
      settings: OpenFcn=warndlg('変更禁止!');
      mask Help: web(ui_blockhelpmng(gcb));
      mask Display: disp('ATC.DSRVEC')
  Block2834 : Outport
  wires:
  Block2832/1 -> SubSystem1082/1(element), SubSystem1081/1(element), SubSystem1080/1(element)
  SubSystem1082/1(Output) -> Block2833/4
  SubSystem1081/1(Output) -> Block2833/3
  SubSystem1080/1(Output) -> Block2833/2
  Block2833/1 -> Block2834/1
  Block2831/1 -> Block2833/1

== system lib_all/SubSystem1023/SubSystem1025/SubSystem1052/SubSystem1060/SubSystem1083/SubSystem1084 ==
  note: ***編集禁止***
  element : Inport
  Inside_DSR : DataStoreRead  DataStoreName=DSM2
  Selector : Selector  IndexOptions=Index vector (port)  Indices=[1 3]  OutputSizes=1
  Output : Outport
  wires:
  Inside_DSR/1 -> Selector/1
  element/1 -> Selector/2
  Selector/1 -> Output/1

== system lib_all/SubSystem1023/SubSystem1025/SubSystem1052/SubSystem1060/SubSystem1083/SubSystem1085 ==
  Block2842 : Inport
  Block2843 : Inport  Port=2
  Block2844 : MultiPortSwitch  zeroidx=off  RndMeth=Zero  SaturateOnIntegerOverflow=on  InputSameDT=on
  SubSystem1086 : Subsystem  TreatAsAtomicUnit=on  mask(***ATC.DSRVEC***){bd_version=1.01.A, r0=DSM18, r1=-1, r2=, r3=, r4=, r5=, r6=, r7=, r8=, r9=, c0=, c1=, c2=, c3=, c4=, c5=, c6=, c7=, c8=, c9=}
      mask prompts: bd_version "Version"
      mask help: これはAutoCからのブロックです．編集禁止．
      settings: OpenFcn=warndlg('変更禁止!');
      mask Help: web(ui_blockhelpmng(gcb));
      mask Display: disp('ATC.DSRVEC')
  SubSystem1087 : Subsystem  TreatAsAtomicUnit=on  mask(***ATC.DSRVEC***){bd_version=1.01.A, r0=DSM8, r1=-1, r2=, r3=, r4=, r5=, r6=, r7=, r8=, r9=, c0=, c1=, c2=, c3=, c4=, c5=, c6=, c7=, c8=, c9=}
      mask prompts: bd_version "Version"
      mask help: これはAutoCからのブロックです．編集禁止．
      settings: OpenFcn=warndlg('変更禁止!');
      mask Help: web(ui_blockhelpmng(gcb));
      mask Display: disp('ATC.DSRVEC')
  SubSystem1088 : Subsystem  TreatAsAtomicUnit=on  mask(***ATC.DSRVEC***){bd_version=1.01.A, r0=DSM11, r1=-1, r2=, r3=, r4=, r5=, r6=, r7=, r8=, r9=, c0=, c1=, c2=, c3=, c4=, c5=, c6=, c7=, c8=, c9=}
      mask prompts: bd_version "Version"
      mask help: これはAutoCからのブロックです．編集禁止．
      settings: OpenFcn=warndlg('変更禁止!');
      mask Help: web(ui_blockhelpmng(gcb));
      mask Display: disp('ATC.DSRVEC')
  Block2845 : Outport
  wires:
  Block2843/1 -> SubSystem1088/1(element), SubSystem1087/1(element), SubSystem1086/1(element)
  SubSystem1088/1(Output) -> Block2844/4
  SubSystem1087/1(Output) -> Block2844/3
  SubSystem1086/1(Output) -> Block2844/2
  Block2844/1 -> Block2845/1
  Block2842/1 -> Block2844/1

== system lib_all/SubSystem1023/SubSystem1025/SubSystem1052/SubSystem1060/SubSystem1083/SubSystem1089 ==
  Block2846 : Inport
  Block2847 : Inport  Port=2
  Block2848 : MultiPortSwitch  zeroidx=off  RndMeth=Zero  SaturateOnIntegerOverflow=on  InputSameDT=on
  SubSystem1090 : Subsystem  TreatAsAtomicUnit=on  mask(***ATC.DSRVEC***){bd_version=1.01.A, r0=DSM18, r1=-1, r2=, r3=, r4=, r5=, r6=, r7=, r8=, r9=, c0=, c1=, c2=, c3=, c4=, c5=, c6=, c7=, c8=, c9=}
      mask prompts: bd_version "Version"
      mask help: これはAutoCからのブロックです．編集禁止．
      settings: OpenFcn=warndlg('変更禁止!');
      mask Help: web(ui_blockhelpmng(gcb));
      mask Display: disp('ATC.DSRVEC')
  SubSystem1091 : Subsystem  TreatAsAtomicUnit=on  mask(***ATC.DSRVEC***){bd_version=1.01.A, r0=DSM8, r1=-1, r2=, r3=, r4=, r5=, r6=, r7=, r8=, r9=, c0=, c1=, c2=, c3=, c4=, c5=, c6=, c7=, c8=, c9=}
      mask prompts: bd_version "Version"
      mask help: これはAutoCからのブロックです．編集禁止．
      settings: OpenFcn=warndlg('変更禁止!');
      mask Help: web(ui_blockhelpmng(gcb));
      mask Display: disp('ATC.DSRVEC')
  SubSystem1092 : Subsystem  TreatAsAtomicUnit=on  mask(***ATC.DSRVEC***){bd_version=1.01.A, r0=DSM11, r1=-1, r2=, r3=, r4=, r5=, r6=, r7=, r8=, r9=, c0=, c1=, c2=, c3=, c4=, c5=, c6=, c7=, c8=, c9=}
      mask prompts: bd_version "Version"
      mask help: これはAutoCからのブロックです．編集禁止．
      settings: OpenFcn=warndlg('変更禁止!');
      mask Help: web(ui_blockhelpmng(gcb));
      mask Display: disp('ATC.DSRVEC')
  Block2849 : Outport
  wires:
  Block2847/1 -> SubSystem1092/1(element), SubSystem1091/1(element), SubSystem1090/1(element)
  SubSystem1092/1(Output) -> Block2848/4
  SubSystem1091/1(Output) -> Block2848/3
  SubSystem1090/1(Output) -> Block2848/2
  Block2848/1 -> Block2849/1
  Block2846/1 -> Block2848/1

== system lib_all/SubSystem1023/SubSystem1093/SubSystem1106/SubSystem1109/SubSystem1110/SubSystem1111 ==
  note: ***編集禁止***
  In1 : Inport
  In2 : Inport  Port=2
  RO1 : RelationalOperator  Operator===  InputSameDT=on
  single1 : DataTypeConversion  OutDataTypeStr=single  SaturateOnIntegerOverflow=on  RndMeth=Zero
  single2 : DataTypeConversion  OutDataTypeStr=single  SaturateOnIntegerOverflow=on  RndMeth=Zero
  Out1 : Outport
  wires:
  single2/1 -> RO1/2
  single1/1 -> RO1/1
  In1/1 -> single1/1
  In2/1 -> single2/1
  RO1/1 -> Out1/1

== system lib_all/SubSystem1023/SubSystem1093/SubSystem1106/SubSystem1109/SubSystem1110/SubSystem1112 ==
  note: ***編集禁止***
  In1 : Inport
  In2 : Inport  Port=2
  RO1 : RelationalOperator  Operator===  InputSameDT=on
  single1 : DataTypeConversion  OutDataTypeStr=single  SaturateOnIntegerOverflow=on  RndMeth=Zero
  single2 : DataTypeConversion  OutDataTypeStr=single  SaturateOnIntegerOverflow=on  RndMeth=Zero
  Out1 : Outport
  wires:
  single2/1 -> RO1/2
  single1/1 -> RO1/1
  In1/1 -> single1/1
  In2/1 -> single2/1
  RO1/1 -> Out1/1

== system lib_all/SubSystem1023/SubSystem1093/SubSystem1106/SubSystem1109/SubSystem1110/SubSystem1113 ==
  Block2949 : Inport
  Block2950 : Inport  Port=2
  Block2951 : Inport  Port=3
  Block2952 : Sum  OutDataTypeStr=double  RndMeth=Zero  SaturateOnIntegerOverflow=on  InputSameDT=on
  Block2953 : Switch  Threshold=0.5  RndMeth=Zero  SaturateOnIntegerOverflow=on  InputSameDT=on
  SubSystem1114 : Subsystem
  Block2954 : Outport
  wires:
  Block2950/1 -> SubSystem1114/1(In1), Block2953/3, Block2952/2
  Block2951/1 -> SubSystem1114/2(In2)
  SubSystem1114/1(Out1) -> Block2953/2
  Block2952/1 -> Block2953/1
  Block2953/1 -> Block2954/1
  Block2949/1 -> Block2952/1

== system lib_all/SubSystem1023/SubSystem1093/SubSystem1106/SubSystem1109/SubSystem1115/SubSystem1116 ==
  note: ***編集禁止***
  In1 : Inport
  In2 : Inport  Port=2
  LO1 : Logic
  RO1 : RelationalOperator  Operator=<  InputSameDT=on
  RO2 : RelationalOperator  Operator=~=  InputSameDT=on
  single1 : DataTypeConversion  OutDataTypeStr=single  SaturateOnIntegerOverflow=on  RndMeth=Zero
  single2 : DataTypeConversion  OutDataTypeStr=single  SaturateOnIntegerOverflow=on  RndMeth=Zero
  Out1 : Outport
  wires:
  LO1/1 -> Out1/1
  RO2/1 -> LO1/2
  RO1/1 -> LO1/1
  single2/1 -> RO2/2, RO1/2
  single1/1 -> RO2/1, RO1/1
  In1/1 -> single1/1
  In2/1 -> single2/1

== system lib_all/SubSystem1023/SubSystem1093/SubSystem1120/SubSystem1122/SubSystem1123/Product ==
  note: ***編集禁止***
  mult : Inport
  div : Inport  Port=2
  init : Inport  Port=3
  Constant1 : Constant  OutDataTypeStr=Inherit: Inherit via back propagation
  Constant2 : Constant  Value=0  OutDataTypeStr=Inherit: Inherit via back propagation
  Product : Product  Inputs=*/  SaturateOnIntegerOverflow=on  RndMeth=Zero  InputSameDT=on  OutDataTypeStr=Inherit: Same as first input
  Relational Operator : RelationalOperator  Operator=~=  InputSameDT=on
  Switch : Switch  Threshold=0.5  SaturateOnIntegerOverflow=on  InputSameDT=on
  Switch1 : Switch  Threshold=0.5  SaturateOnIntegerOverflow=on  InputSameDT=on
  Out : Outport
  wires:
  mult/1 -> Product/1
  Product/1 -> Switch/1
  Relational Operator/1 -> Switch/2, Switch1/2
  init/1 -> Switch/3
  Switch/1 -> Out/1
  div/1 -> Switch1/1, Relational Operator/1
  Switch1/1 -> Product/2
  Constant2/1 -> Relational Operator/2
  Constant1/1 -> Switch1/3

== system lib_all/SubSystem1023/SubSystem1093/SubSystem1120/SubSystem1122/SubSystem1125/SubSystem1126 ==
  note: ***編集禁止***
  In1 : Inport
  In2 : Inport  Port=2
  RO1 : RelationalOperator  Operator=>  InputSameDT=on
  single1 : DataTypeConversion  OutDataTypeStr=single  SaturateOnIntegerOverflow=on  RndMeth=Zero
  single2 : DataTypeConversion  OutDataTypeStr=single  SaturateOnIntegerOverflow=on  RndMeth=Zero
  Out1 : Outport
  wires:
  single2/1 -> RO1/2
  single1/1 -> RO1/1
  In1/1 -> single1/1
  In2/1 -> single2/1
  RO1/1 -> Out1/1

== system lib_all/SubSystem1023/SubSystem1093/SubSystem1120/SubSystem1122/SubSystem1125/SubSystem1127 ==
  note: ***編集禁止***
  In1 : Inport
  In2 : Inport  Port=2
  LO1 : Logic
  RO1 : RelationalOperator  Operator=<  InputSameDT=on
  RO2 : RelationalOperator  Operator=~=  InputSameDT=on
  single1 : DataTypeConversion  OutDataTypeStr=single  SaturateOnIntegerOverflow=on  RndMeth=Zero
  single2 : DataTypeConversion  OutDataTypeStr=single  SaturateOnIntegerOverflow=on  RndMeth=Zero
  Out1 : Outport
  wires:
  LO1/1 -> Out1/1
  RO2/1 -> LO1/2
  RO1/1 -> LO1/1
  single2/1 -> RO2/2, RO1/2
  single1/1 -> RO2/1, RO1/1
  In1/1 -> single1/1
  In2/1 -> single2/1

== system lib_all/SubSystem1023/SubSystem1093/SubSystem1120/SubSystem1128/SubSystem1132/SubSystem1133 ==
  Block3037 : Inport
  Block3038 : Inport  Port=2
  Block3039 : MultiPortSwitch  zeroidx=off  RndMeth=Zero  SaturateOnIntegerOverflow=on  InputSameDT=on
  SubSystem1134 : Subsystem  TreatAsAtomicUnit=on  mask(***ATC.DSRVEC***){bd_version=1.01.A, r0=DSM18, r1=-1, r2=, r3=, r4=, r5=, r6=, r7=, r8=, r9=, c0=, c1=, c2=, c3=, c4=, c5=, c6=, c7=, c8=, c9=}
      mask prompts: bd_version "Version"
      mask help: これはAutoCからのブロックです．編集禁止．
      settings: OpenFcn=warndlg('変更禁止!');
      mask Help: web(ui_blockhelpmng(gcb));
      mask Display: disp('ATC.DSRVEC')
  SubSystem1135 : Subsystem  TreatAsAtomicUnit=on  mask(***ATC.DSRVEC***){bd_version=1.01.A, r0=DSM8, r1=-1, r2=, r3=, r4=, r5=, r6=, r7=, r8=, r9=, c0=, c1=, c2=, c3=, c4=, c5=, c6=, c7=, c8=, c9=}
      mask prompts: bd_version "Version"
      mask help: これはAutoCからのブロックです．編集禁止．
      settings: OpenFcn=warndlg('変更禁止!');
      mask Help: web(ui_blockhelpmng(gcb));
      mask Display: disp('ATC.DSRVEC')
  SubSystem1136 : Subsystem  TreatAsAtomicUnit=on  mask(***ATC.DSRVEC***){bd_version=1.01.A, r0=DSM11, r1=-1, r2=, r3=, r4=, r5=, r6=, r7=, r8=, r9=, c0=, c1=, c2=, c3=, c4=, c5=, c6=, c7=, c8=, c9=}
      mask prompts: bd_version "Version"
      mask help: これはAutoCからのブロックです．編集禁止．
      settings: OpenFcn=warndlg('変更禁止!');
      mask Help: web(ui_blockhelpmng(gcb));
      mask Display: disp('ATC.DSRVEC')
  Block3040 : Outport
  wires:
  Block3038/1 -> SubSystem1136/1(element), SubSystem1135/1(element), SubSystem1134/1(element)
  SubSystem1136/1(Output) -> Block3039/4
  SubSystem1135/1(Output) -> Block3039/3
  SubSystem1134/1(Output) -> Block3039/2
  Block3039/1 -> Block3040/1
  Block3037/1 -> Block3039/1
